simulink model "AirDirecCtrlMgr"  (R2022b)
settings: Solver=FixedStepDiscrete  StartTime=0.0  StopTime=10.0  FixedStep=0.2  RelTol=1e-3
(part 2 of 2)

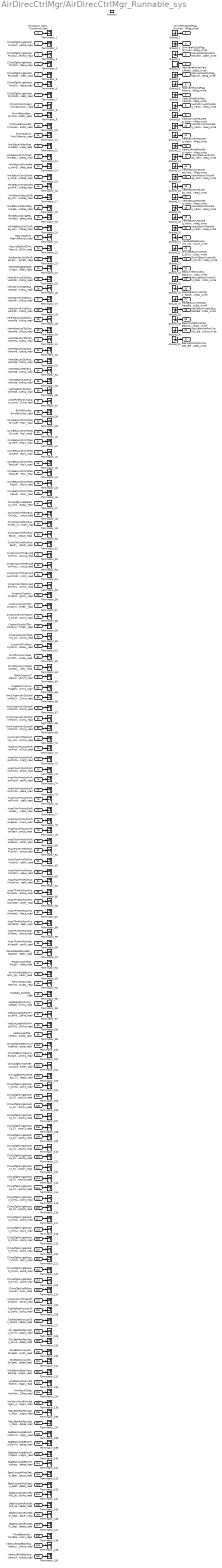
== system AirDirecCtrlMgr/AirDirecCtrlMgr_Runnable_sys ==
  ClimaActv_Ngcc_ClimaActv_read : Inport
  ClimaCfgForVgaMotorPrsntAtRowFirstLeMid_ClimaCfgForVgaMotorPrsntAtRowFirstLeMid_read : Inport  Port=2
  ClimaCfgForVgaMotorPrsntAtRowFirstRiMid_ClimaCfgForVgaMotorPrsntAtRowFirstRiMid_read : Inport  Port=3
  ClimaCfgForVgaMotorPrsntAtRowFirstSideLe_ClimaCfgForVgaMotorPrsntAtRowFirstSideLe_read : Inport  Port=4
  ClimaCfgForVgaMotorPrsntAtRowFirstSideRi_ClimaCfgForVgaMotorPrsntAtRowFirstSideRi_read : Inport  Port=5
  ClimaCfgForVgaMotorPrsntAtRowSecSideLe_ClimaCfgForVgaMotorPrsntAtRowSecSideLe_read : Inport  Port=6
  ClimaCfgForVgaMotorPrsntAtRowSecSideRi_ClimaCfgForVgaMotorPrsntAtRowSecSideRi_read : Inport  Port=7
  ClimaCtrlActvNgcc_ClimaCtrlActv_read : Inport  Port=8
  DrvrHdPosnDetn_DrvrHdPosnDetn_read : Inport  Port=9
  FrntHvacBlowerSts_FrntHvacBlowerSts_read : Inport  Port=10
  FrntVGAerror_FrntVGAerror_read : Inport  Port=11
  HmiElecAirAdpvReq_HmiElecAirAdpvReq_read : Inport  Port=12
  HmiElecAirDirCrtlReq_HmiElecAirDirCrtlReq_read : Inport  Port=13
  HmiElecAirDirModReq_HmiElecAirDirModReq_read : Inport  Port=14
  HmiElecAirDrvrSwtReq_HmiElecAirDrvrSwtReq_read : Inport  Port=15
  HmiElecAirFrntSwtReq_HmiElecAirFrntSwtReq_read : Inport  Port=16
  HmiElecAirPassSwtReq_HmiElecAirPassSwtReq_read : Inport  Port=17
  HmiElecAirReSwtReq_HmiElecAirReSwtReq_read : Inport  Port=18
  HmiElecAirSwngReq_HmiElecAirSwngReq_read : Inport  Port=19
  HmiReElecAirDirCrtlReq_HmiReElecAirDirCrtlReq_read : Inport  Port=20
  RearVGAerror_RearVGAerror_read : Inport  Port=21
  ResrvdSigForECM4_ResrvdSigForECM4_read : Inport  Port=22
  StrtRemSts1WdStrtRemSts_Ngcc_StrtRemSts1WdStrtRemSts_read : Inport  Port=23
  VehModMngtGlbSafe1_Ngcc_VehModMngtGlbSafe1_read : Inport  Port=24
  VentnDrvrLeXStsRsp_VentnDrvrLeXStsRsp_read : Inport  Port=25
  VentnDrvrLeYStsRsp_VentnDrvrLeYStsRsp_read : Inport  Port=26
  VentnDrvrRiXStsRsp_VentnDrvrRiXStsRsp_read : Inport  Port=27
  VentnDrvrRiYStsRsp_VentnDrvrRiYStsRsp_read : Inport  Port=28
  VentnPassLeXStsRsp_VentnPassLeXStsRsp_read : Inport  Port=29
  VentnPassLeYStsRsp_VentnPassLeYStsRsp_read : Inport  Port=30
  VentnPassRiXStsRsp_VentnPassRiXStsRsp_read : Inport  Port=31
  VentnPassRiYStsRsp_VentnPassRiYStsRsp_read : Inport  Port=32
  VentnSecLeXStsRsp_VentnSecLeXStsRsp_read : Inport  Port=33
  VentnSecLeYStsRsp_VentnSecLeYStsRsp_read : Inport  Port=34
  VentnSecRiXStsRsp_VentnSecRiXStsRsp_read : Inport  Port=35
  VentnSecRiYStsRsp_VentnSecRiYStsRsp_read : Inport  Port=36
  AmbTFildForClimaNgcc_AmbTFildForClima_read : Inport  Port=37
  BlwAftRunSts_BlwAftRunSts_read : Inport  Port=38
  CcmElecAirDirCrtlReqDrvrLePosX_CcmElecAirDirCrtlReqDrvrLePosX_read : Inport  Port=39
  CcmElecAirDirCrtlReqDrvrLePosY_CcmElecAirDirCrtlReqDrvrLePosY_read : Inport  Port=40
  CcmElecAirDirCrtlReqDrvrRiPosX_CcmElecAirDirCrtlReqDrvrRiPosX_read : Inport  Port=41
  CcmElecAirDirCrtlReqDrvrRiPosY_CcmElecAirDirCrtlReqDrvrRiPosY_read : Inport  Port=42
  CcmElecAirDirCrtlReqPassLePosX_CcmElecAirDirCrtlReqPassLePosX_read : Inport  Port=43
  CcmElecAirDirCrtlReqPassLePosY_CcmElecAirDirCrtlReqPassLePosY_read : Inport  Port=44
  CcmElecAirDirCrtlReqPassRiPosX_CcmElecAirDirCrtlReqPassRiPosX_read : Inport  Port=45
  CcmElecAirDirCrtlReqPassRiPosY_CcmElecAirDirCrtlReqPassRiPosY_read : Inport  Port=46
  ClimaCfgForHeadRadar_ClimaCfgForHeadRadar_read : Inport  Port=47
  ClimaTAtCmftForRowFirstLe_ClimaTAtCmftForRowFirstLe_read : Inport  Port=48
  ClimaTAtCmftForRowFirstRi_ClimaTAtCmftForRowFirstRi_read : Inport  Port=49
  ClimaTAtCmftForRowSecLe_ClimaTAtCmftForRowSecLe_read : Inport  Port=50
  ClimaTAtCmftForRowSecRi_ClimaTAtCmftForRowSecRi_read : Inport  Port=51
  CmptmtAirTCtrlErrAtRowFirstAvg_CmptmtAirTCtrlErrAtRowFirstAvg_read : Inport  Port=52
  CmptmtAirTCtrlErrAtRowFirstLe_CmptmtAirTCtrlErrAtRowFirstLe_read : Inport  Port=53
  CmptmtAirTCtrlErrAtRowFirstRi_CmptmtAirTCtrlErrAtRowFirstRi_read : Inport  Port=54
  CmptmtAirTEstimdAtRowFirst_CmptmtAirTEstimdAtRowFirst_read : Inport  Port=55
  CmptmtClngActv_CmptmtClngActv_read : Inport  Port=56
  CmptmtIntrMtrlTErr_CmptmtIntrMtrlTErr_read : Inport  Port=57
  CmptmtIntrMtrlTEstimd_CmptmtIntrMtrlTEstimd_read : Inport  Port=58
  CmptmtSunMtrlTErr_CmptmtSunMtrlTErr_read : Inport  Port=59
  CmptmtSunMtrlTEstimd_CmptmtSunMtrlTEstimd_read : Inport  Port=60
  CmptmtTFrntRaw_CmptmtTFrntRaw_read : Inport  Port=61
  DrvrPfmncAlrmReq_DrvrPfmncAlrmReq_read : Inport  Port=62
  DrvrPfmncAlrmReqFromDHU_DrvrPfmncAlrmReqFromDHU_read : Inport  Port=63
  FbForCmptmtT_FbForCmptmtT_read : Inport  Port=64
  FragStsFrmClima_FragStsFrmClima_read : Inport  Port=65
  HmiCmptmtAirDistbnFrntForClima_HmiCmptmtAirDistbnFrntForClima_read : Inport  Port=66
  HmiCmptmtAirDistbnFrntForClima_HmiCmptmtAirDistbnFrntRiForClima_read : Inport  Port=67
  HmiCmptmtAirDistbnFrntForClima_HmiCmptmtAirDistbnReForClima_read : Inport  Port=68
  HmiCmptmtAirDistbnFrntForClima_HmiCmptmtAirDistbnReRiForClima_read : Inport  Port=69
  HmiCmptmtTSpForClima_HmiCmptmtTSpForClima_read : Inport  Port=70
  HvacFlowFrntActForRowFirst_HvacFlowFrntActForRowFirst_read : Inport  Port=71
  HvacFlowFrntActForRowFirstAtVent_HvacFlowFrntActForRowFirstAtVent_read : Inport  Port=72
  HvacFlowFrntActForRowFirstAtVent_HvacFlowFrntActForRowFirstAtVentLe_read : Inport  Port=73
  HvacFlowFrntActForRowFirstAtVent_HvacFlowFrntActForRowFirstAtVentRi_read : Inport  Port=74
  HvacFlowFrntActForRowFirstAtVent_HvacFlowFrntActForRowFirstAtVentSideLe_read : Inport  Port=75
  HvacFlowFrntActForRowFirstAtVent_HvacFlowFrntActForRowFirstAtVentSideRi_read : Inport  Port=76
  HvacFlowFrntActForRowSec_HvacFlowFrntActForRowSec_read : Inport  Port=77
  HvacFlowFrntActForRowSecAtVent_HvacFlowFrntActForRowSecAtVent_read : Inport  Port=78
  HvacFlowFrntActForRowSecAtVent_HvacFlowFrntActForRowSecAtVentLe_read : Inport  Port=79
  HvacFlowFrntActForRowSecAtVent_HvacFlowFrntActForRowSecAtVentRi_read : Inport  Port=80
  HvacFlowFrntForRowFirstAtVentLe_HvacFlowFrntForRowFirstAtVentLe_read : Inport  Port=81
  HvacFlowFrntForRowFirstAtVentRi_HvacFlowFrntForRowFirstAtVentRi_read : Inport  Port=82
  HvacFlowFrntForRowFirstAtVentSideLe_HvacFlowFrntForRowFirstAtVentSideLe_read : Inport  Port=83
  HvacFlowFrntForRowFirstAtVentSideRi_HvacFlowFrntForRowFirstAtVentSideRi_read : Inport  Port=84
  HvacTFrntForRowFirstAtVentLe_HvacTFrntForRowFirstAtVentLe_read : Inport  Port=85
  HvacTFrntForRowFirstAtVentRi_HvacTFrntForRowFirstAtVentRi_read : Inport  Port=86
  HvacTFrntForRowFirstAtVentSideLe_HvacTFrntForRowFirstAtVentSideLe_read : Inport  Port=87
  HvacTFrntForRowFirstAtVentSideRi_HvacTFrntForRowFirstAtVentSideRi_read : Inport  Port=88
  HvacTFrntForRowSecAtVentLe_HvacTFrntForRowSecAtVentLe_read : Inport  Port=89
  HvacTFrntForRowSecAtVentRi_HvacTFrntForRowSecAtVentRi_read : Inport  Port=90
  PassSideHdPosnDetn_PassSideHdPosnDetn_read : Inport  Port=91
  PrkgClimaOnReq_PrkgClimaOnReq_read : Inport  Port=92
  PwrAllwdForElecAirVentn_Ngcc_PwrAllwdForElecAirVentn_read : Inport  Port=93
  RemVentActvSts_RemVentActvSts_read : Inport  Port=94
  SunData_SunData_read : Inport  Port=95
  VehSpdLgtForClima_VehSpdLgtForClima_read : Inport  Port=96
  VehSunLoadAtRowFirstLeFild_VehSunLoadAtRowFirstLeFild_read : Inport  Port=97
  VehSunLoadAtRowFirstRiFild_VehSunLoadAtRowFirstRiFild_read : Inport  Port=98
  VehSunLoadTot_VehSunLoadTot_read : Inport  Port=99
  ClimaCfgForElectricLiftingModule_ClimaCfgForElectricLiftingModule_read : Inport  Port=100
  ClimaCfgForInteriorViewCamera_ClimaCfgForInteriorViewCamera_read : Inport  Port=101
  ClimaCfgForMARKET_ClimaCfgForMARKET_read : Inport  Port=102
  ClimaCfgForPowerSeats_ClimaCfgForPowerSeats_read : Inport  Port=103
  ClimaCfgForVgaMotor1_ClimaCfgForVgaMotor1_read : Inport  Port=104
  ClimaCfgForVgaMotor10_ClimaCfgForVgaMotor10_read : Inport  Port=105
  ClimaCfgForVgaMotor11_ClimaCfgForVgaMotor11_read : Inport  Port=106
  ClimaCfgForVgaMotor12_ClimaCfgForVgaMotor12_read : Inport  Port=107
  ClimaCfgForVgaMotor13_ClimaCfgForVgaMotor13_read : Inport  Port=108
  ClimaCfgForVgaMotor14_ClimaCfgForVgaMotor14_read : Inport  Port=109
  ClimaCfgForVgaMotor15_ClimaCfgForVgaMotor15_read : Inport  Port=110
  ClimaCfgForVgaMotor16_ClimaCfgForVgaMotor16_read : Inport  Port=111
  ClimaCfgForVgaMotor17_ClimaCfgForVgaMotor17_read : Inport  Port=112
  ClimaCfgForVgaMotor18_ClimaCfgForVgaMotor18_read : Inport  Port=113
  ClimaCfgForVgaMotor19_ClimaCfgForVgaMotor19_read : Inport  Port=114
  ClimaCfgForVgaMotor2_ClimaCfgForVgaMotor2_read : Inport  Port=115
  ClimaCfgForVgaMotor20_ClimaCfgForVgaMotor20_read : Inport  Port=116
  ClimaCfgForVgaMotor3_ClimaCfgForVgaMotor3_read : Inport  Port=117
  ClimaCfgForVgaMotor4_ClimaCfgForVgaMotor4_read : Inport  Port=118
  ClimaCfgForVgaMotor5_ClimaCfgForVgaMotor5_read : Inport  Port=119
  ClimaCfgForVgaMotor6_ClimaCfgForVgaMotor6_read : Inport  Port=120
  ClimaCfgForVgaMotor7_ClimaCfgForVgaMotor7_read : Inport  Port=121
  ClimaCfgForVgaMotor8_ClimaCfgForVgaMotor8_read : Inport  Port=122
  ClimaCfgForVgaMotor9_ClimaCfgForVgaMotor9_read : Inport  Port=123
  ClimaCfgWindFollowHumanMov_ClimaCfgWindFollowHumanMov_read : Inport  Port=124
  CmptmtAirTCtrlErrQf_CmptmtAirTCtrlErrQf_read : Inport  Port=125
  CopilotSeatOccupySts_CopilotSeatOccupySts_read : Inport  Port=126
  CopilotSeatOccupySts_CopilotSeatOccupySts_IsUpdated_read : Inport  Port=127
  DrvrSeatPosPercNgcc_DrvrSeatPosPercNgcc_read : Inport  Port=128
  DrvrSeatPosPercNgcc_DrvrSeatPosPercNgcc_IsUpdated_read : Inport  Port=129
  DrvSeatOccupySts_DrvSeatOccupySts_read : Inport  Port=130
  DrvSeatOccupySts_DrvSeatOccupySts_IsUpdated_read : Inport  Port=131
  HmiElecAirBodyVentLebtnNgcc_HmiElecAirBodyVentLebtnNgcc_read : Inport  Port=132
  HmiElecAirBodyVentRibtnNgcc_HmiElecAirBodyVentRibtnNgcc_read : Inport  Port=133
  HmiMaxACReq_HmiMaxACReq_read : Inport  Port=134
  HmiSmrtWindFolwSetNgcc_HmiSmrtWindFolwSetNgcc_read : Inport  Port=135
  PassSeatPosPercNgcc_PassSeatPosPercNgcc_read : Inport  Port=136
  PassSeatPosPercNgcc_PassSeatPosPercNgcc_IsUpdated_read : Inport  Port=137
  SeatBackAngleRowFirstDrvrNgcc_SeatBackAngleRowFirstDrvrNgcc_read : Inport  Port=138
  SeatBackAngleRowFirstDrvrNgcc_SeatBackAngleRowFirstDrvrNgcc_IsUpdated_read : Inport  Port=139
  SeatBackAngleRowFirstPassNgcc_SeatBackAngleRowFirstPassNgcc_read : Inport  Port=140
  SeatBackAngleRowFirstPassNgcc_SeatBackAngleRowFirstPassNgcc_IsUpdated_read : Inport  Port=141
  SeatOccptAtRowSecLe_SeatOccptAtRowSecLe_read : Inport  Port=142
  SeatOccptAtRowSecLe_SeatOccptAtRowSecLe_IsUpdated_read : Inport  Port=143
  SeatOccptAtRowSecMid_SeatOccptAtRowSecMid_read : Inport  Port=144
  SeatOccptAtRowSecMid_SeatOccptAtRowSecMid_IsUpdated_read : Inport  Port=145
  SeatOccptAtRowSecRi_SeatOccptAtRowSecRi_read : Inport  Port=146
  SeatOccptAtRowSecRi_SeatOccptAtRowSecRi_IsUpdated_read : Inport  Port=147
  TiAndDateIndcn_TiAndDateIndcn_read : Inport  Port=148
  VentnLiftModPosnRsp_VentnLiftModPosnRsp_read : Inport  Port=149
  VentnLiftModStsRsp_VentnLiftModStsRsp_read : Inport  Port=150
  function : TriggerPort  TriggerType=function-call  VariantControl=(inherit)  SampleTimeType=periodic  SampleTime=-1
  Ground_{1..3,5..31} (30 alike) : Ground
  Ground_4 : Subsystem
  Terminator_{1..150} (150 alike) : Terminator
  DrvrVentLeClsdFlag_DrvrVentLeClsdFlag_write : Outport
      settings: EnsureOutportIsVirtual=on
  DrvrVentRiClsdFlag_DrvrVentRiClsdFlag_write : Outport  Port=2
      settings: EnsureOutportIsVirtual=on
  MemSaveFlgForCenVentLiftModErr_MemSaveFlgForCenVentLiftModErr_write : Outport  Port=3
      settings: EnsureOutportIsVirtual=on
  MemSaveForCenVentLiftModSts_MemSaveForCenVentLiftModSts_write : Outport  Port=4
      settings: EnsureOutportIsVirtual=on
  PassVentLeClsdFlag_PassVentLeClsdFlag_write : Outport  Port=5
      settings: EnsureOutportIsVirtual=on
  PassVentRiClsdFlag_PassVentRiClsdFlag_write : Outport  Port=6
      settings: EnsureOutportIsVirtual=on
  VentLiftModCaliReq_VentLiftModCaliReq_write : Outport  Port=7
      settings: EnsureOutportIsVirtual=on
  VentnDrvrLeXPsonReq_VentnDrvrLeXPsonReq_write : Outport  Port=8
      settings: EnsureOutportIsVirtual=on
  VentnDrvrLeYPsonReq_VentnDrvrLeYPsonReq_write : Outport  Port=9
      settings: EnsureOutportIsVirtual=on
  VentnDrvrRiXPsonReq_VentnDrvrRiXPsonReq_write : Outport  Port=10
      settings: EnsureOutportIsVirtual=on
  VentnDrvrRiYPsonReq_VentnDrvrRiYPsonReq_write : Outport  Port=11
      settings: EnsureOutportIsVirtual=on
  VentnLiftModPosnReq_VentnLiftModPosnReq_write : Outport  Port=12
      settings: EnsureOutportIsVirtual=on
  VentnPassLeXPsonReq_VentnPassLeXPsonReq_write : Outport  Port=13
      settings: EnsureOutportIsVirtual=on
  VentnPassLeYPsonReq_VentnPassLeYPsonReq_write : Outport  Port=14
      settings: EnsureOutportIsVirtual=on
  VentnPassRiXPsonReq_VentnPassRiXPsonReq_write : Outport  Port=15
      settings: EnsureOutportIsVirtual=on
  VentnPassRiYPsonReq_VentnPassRiYPsonReq_write : Outport  Port=16
      settings: EnsureOutportIsVirtual=on
  VentnSecLeXPosnReq_VentnSecLeXPosnReq_write : Outport  Port=17
      settings: EnsureOutportIsVirtual=on
  VentnSecLeYPosnReq_VentnSecLeYPosnReq_write : Outport  Port=18
      settings: EnsureOutportIsVirtual=on
  VentnSecRiXPosnReq_VentnSecRiXPosnReq_write : Outport  Port=19
      settings: EnsureOutportIsVirtual=on
  VentnSecRiYPosnReq_VentnSecRiYPosnReq_write : Outport  Port=20
      settings: EnsureOutportIsVirtual=on
  VGAStopDuePowerLimit_VGAStopDuePowerLimit_write : Outport  Port=21
      settings: EnsureOutportIsVirtual=on
  DrvrLeElecAirVentnSts_DrvrLeElecAirVentnSts_write : Outport  Port=22
      settings: EnsureOutportIsVirtual=on
  DrvrRiElecAirVentnSts_DrvrRiElecAirVentnSts_write : Outport  Port=23
      settings: EnsureOutportIsVirtual=on
  ElecAirVentnAvlSts_ElecAirVentnAvlSts_write : Outport  Port=24
      settings: EnsureOutportIsVirtual=on
  PassLeElecAirVentnSts_PassLeElecAirVentnSts_write : Outport  Port=25
      settings: EnsureOutportIsVirtual=on
  PassRiElecAirVentnSts_PassRiElecAirVentnSts_write : Outport  Port=26
      settings: EnsureOutportIsVirtual=on
  ReLeElecAirVentnSts_ReLeElecAirVentnSts_write : Outport  Port=27
      settings: EnsureOutportIsVirtual=on
  ReRiElecAirVentnSts_ReRiElecAirVentnSts_write : Outport  Port=28
      settings: EnsureOutportIsVirtual=on
  EleAirOutletCmpFact_EleAirOutletCmpFact_write : Outport  Port=29
      settings: EnsureOutportIsVirtual=on
  ElecVentnMotPwrCnsAct_ElecVentnMotPwrCnsAct_write : Outport  Port=30
      settings: EnsureOutportIsVirtual=on
  ElecVentnMotPwrCnsDes_ElecVentnMotPwrCnsDes_write : Outport  Port=31
      settings: EnsureOutportIsVirtual=on
  wires:
  ClimaActv_Ngcc_ClimaActv_read/1 -> Terminator_1/1
  ClimaCfgForVgaMotorPrsntAtRowFirstLeMid_ClimaCfgForVgaMotorPrsntAtRowFirstLeMid_read/1 -> Terminator_2/1
  ClimaCfgForVgaMotorPrsntAtRowFirstRiMid_ClimaCfgForVgaMotorPrsntAtRowFirstRiMid_read/1 -> Terminator_3/1
  ClimaCfgForVgaMotorPrsntAtRowFirstSideLe_ClimaCfgForVgaMotorPrsntAtRowFirstSideLe_read/1 -> Terminator_4/1
  ClimaCfgForVgaMotorPrsntAtRowFirstSideRi_ClimaCfgForVgaMotorPrsntAtRowFirstSideRi_read/1 -> Terminator_5/1
  ClimaCfgForVgaMotorPrsntAtRowSecSideLe_ClimaCfgForVgaMotorPrsntAtRowSecSideLe_read/1 -> Terminator_6/1
  ClimaCfgForVgaMotorPrsntAtRowSecSideRi_ClimaCfgForVgaMotorPrsntAtRowSecSideRi_read/1 -> Terminator_7/1
  ClimaCtrlActvNgcc_ClimaCtrlActv_read/1 -> Terminator_8/1
  DrvrHdPosnDetn_DrvrHdPosnDetn_read/1 -> Terminator_9/1
  FrntHvacBlowerSts_FrntHvacBlowerSts_read/1 -> Terminator_10/1
  FrntVGAerror_FrntVGAerror_read/1 -> Terminator_11/1
  HmiElecAirAdpvReq_HmiElecAirAdpvReq_read/1 -> Terminator_12/1
  HmiElecAirDirCrtlReq_HmiElecAirDirCrtlReq_read/1 -> Terminator_13/1
  HmiElecAirDirModReq_HmiElecAirDirModReq_read/1 -> Terminator_14/1
  HmiElecAirDrvrSwtReq_HmiElecAirDrvrSwtReq_read/1 -> Terminator_15/1
  HmiElecAirFrntSwtReq_HmiElecAirFrntSwtReq_read/1 -> Terminator_16/1
  HmiElecAirPassSwtReq_HmiElecAirPassSwtReq_read/1 -> Terminator_17/1
  HmiElecAirReSwtReq_HmiElecAirReSwtReq_read/1 -> Terminator_18/1
  HmiElecAirSwngReq_HmiElecAirSwngReq_read/1 -> Terminator_19/1
  HmiReElecAirDirCrtlReq_HmiReElecAirDirCrtlReq_read/1 -> Terminator_20/1
  RearVGAerror_RearVGAerror_read/1 -> Terminator_21/1
  ResrvdSigForECM4_ResrvdSigForECM4_read/1 -> Terminator_22/1
  StrtRemSts1WdStrtRemSts_Ngcc_StrtRemSts1WdStrtRemSts_read/1 -> Terminator_23/1
  VehModMngtGlbSafe1_Ngcc_VehModMngtGlbSafe1_read/1 -> Terminator_24/1
  VentnDrvrLeXStsRsp_VentnDrvrLeXStsRsp_read/1 -> Terminator_25/1
  VentnDrvrLeYStsRsp_VentnDrvrLeYStsRsp_read/1 -> Terminator_26/1
  VentnDrvrRiXStsRsp_VentnDrvrRiXStsRsp_read/1 -> Terminator_27/1
  VentnDrvrRiYStsRsp_VentnDrvrRiYStsRsp_read/1 -> Terminator_28/1
  VentnPassLeXStsRsp_VentnPassLeXStsRsp_read/1 -> Terminator_29/1
  VentnPassLeYStsRsp_VentnPassLeYStsRsp_read/1 -> Terminator_30/1
  VentnPassRiXStsRsp_VentnPassRiXStsRsp_read/1 -> Terminator_31/1
  VentnPassRiYStsRsp_VentnPassRiYStsRsp_read/1 -> Terminator_32/1
  VentnSecLeXStsRsp_VentnSecLeXStsRsp_read/1 -> Terminator_33/1
  VentnSecLeYStsRsp_VentnSecLeYStsRsp_read/1 -> Terminator_34/1
  VentnSecRiXStsRsp_VentnSecRiXStsRsp_read/1 -> Terminator_35/1
  VentnSecRiYStsRsp_VentnSecRiYStsRsp_read/1 -> Terminator_36/1
  AmbTFildForClimaNgcc_AmbTFildForClima_read/1 -> Terminator_37/1
  BlwAftRunSts_BlwAftRunSts_read/1 -> Terminator_38/1
  CcmElecAirDirCrtlReqDrvrLePosX_CcmElecAirDirCrtlReqDrvrLePosX_read/1 -> Terminator_39/1
  CcmElecAirDirCrtlReqDrvrLePosY_CcmElecAirDirCrtlReqDrvrLePosY_read/1 -> Terminator_40/1
  CcmElecAirDirCrtlReqDrvrRiPosX_CcmElecAirDirCrtlReqDrvrRiPosX_read/1 -> Terminator_41/1
  CcmElecAirDirCrtlReqDrvrRiPosY_CcmElecAirDirCrtlReqDrvrRiPosY_read/1 -> Terminator_42/1
  CcmElecAirDirCrtlReqPassLePosX_CcmElecAirDirCrtlReqPassLePosX_read/1 -> Terminator_43/1
  CcmElecAirDirCrtlReqPassLePosY_CcmElecAirDirCrtlReqPassLePosY_read/1 -> Terminator_44/1
  CcmElecAirDirCrtlReqPassRiPosX_CcmElecAirDirCrtlReqPassRiPosX_read/1 -> Terminator_45/1
  CcmElecAirDirCrtlReqPassRiPosY_CcmElecAirDirCrtlReqPassRiPosY_read/1 -> Terminator_46/1
  ClimaCfgForHeadRadar_ClimaCfgForHeadRadar_read/1 -> Terminator_47/1
  ClimaTAtCmftForRowFirstLe_ClimaTAtCmftForRowFirstLe_read/1 -> Terminator_48/1
  ClimaTAtCmftForRowFirstRi_ClimaTAtCmftForRowFirstRi_read/1 -> Terminator_49/1
  ClimaTAtCmftForRowSecLe_ClimaTAtCmftForRowSecLe_read/1 -> Terminator_50/1
  ClimaTAtCmftForRowSecRi_ClimaTAtCmftForRowSecRi_read/1 -> Terminator_51/1
  CmptmtAirTCtrlErrAtRowFirstAvg_CmptmtAirTCtrlErrAtRowFirstAvg_read/1 -> Terminator_52/1
  CmptmtAirTCtrlErrAtRowFirstLe_CmptmtAirTCtrlErrAtRowFirstLe_read/1 -> Terminator_53/1
  CmptmtAirTCtrlErrAtRowFirstRi_CmptmtAirTCtrlErrAtRowFirstRi_read/1 -> Terminator_54/1
  CmptmtAirTEstimdAtRowFirst_CmptmtAirTEstimdAtRowFirst_read/1 -> Terminator_55/1
  CmptmtClngActv_CmptmtClngActv_read/1 -> Terminator_56/1
  CmptmtIntrMtrlTErr_CmptmtIntrMtrlTErr_read/1 -> Terminator_57/1
  CmptmtIntrMtrlTEstimd_CmptmtIntrMtrlTEstimd_read/1 -> Terminator_58/1
  CmptmtSunMtrlTErr_CmptmtSunMtrlTErr_read/1 -> Terminator_59/1
  CmptmtSunMtrlTEstimd_CmptmtSunMtrlTEstimd_read/1 -> Terminator_60/1
  CmptmtTFrntRaw_CmptmtTFrntRaw_read/1 -> Terminator_61/1
  DrvrPfmncAlrmReq_DrvrPfmncAlrmReq_read/1 -> Terminator_62/1
  DrvrPfmncAlrmReqFromDHU_DrvrPfmncAlrmReqFromDHU_read/1 -> Terminator_63/1
  FbForCmptmtT_FbForCmptmtT_read/1 -> Terminator_64/1
  FragStsFrmClima_FragStsFrmClima_read/1 -> Terminator_65/1
  HmiCmptmtAirDistbnFrntForClima_HmiCmptmtAirDistbnFrntForClima_read/1 -> Terminator_66/1
  HmiCmptmtAirDistbnFrntForClima_HmiCmptmtAirDistbnFrntRiForClima_read/1 -> Terminator_67/1
  HmiCmptmtAirDistbnFrntForClima_HmiCmptmtAirDistbnReForClima_read/1 -> Terminator_68/1
  HmiCmptmtAirDistbnFrntForClima_HmiCmptmtAirDistbnReRiForClima_read/1 -> Terminator_69/1
  HmiCmptmtTSpForClima_HmiCmptmtTSpForClima_read/1 -> Terminator_70/1
  HvacFlowFrntActForRowFirst_HvacFlowFrntActForRowFirst_read/1 -> Terminator_71/1
  HvacFlowFrntActForRowFirstAtVent_HvacFlowFrntActForRowFirstAtVent_read/1 -> Terminator_72/1
  HvacFlowFrntActForRowFirstAtVent_HvacFlowFrntActForRowFirstAtVentLe_read/1 -> Terminator_73/1
  HvacFlowFrntActForRowFirstAtVent_HvacFlowFrntActForRowFirstAtVentRi_read/1 -> Terminator_74/1
  HvacFlowFrntActForRowFirstAtVent_HvacFlowFrntActForRowFirstAtVentSideLe_read/1 -> Terminator_75/1
  HvacFlowFrntActForRowFirstAtVent_HvacFlowFrntActForRowFirstAtVentSideRi_read/1 -> Terminator_76/1
  HvacFlowFrntActForRowSec_HvacFlowFrntActForRowSec_read/1 -> Terminator_77/1
  HvacFlowFrntActForRowSecAtVent_HvacFlowFrntActForRowSecAtVent_read/1 -> Terminator_78/1
  HvacFlowFrntActForRowSecAtVent_HvacFlowFrntActForRowSecAtVentLe_read/1 -> Terminator_79/1
  HvacFlowFrntActForRowSecAtVent_HvacFlowFrntActForRowSecAtVentRi_read/1 -> Terminator_80/1
  HvacFlowFrntForRowFirstAtVentLe_HvacFlowFrntForRowFirstAtVentLe_read/1 -> Terminator_81/1
  HvacFlowFrntForRowFirstAtVentRi_HvacFlowFrntForRowFirstAtVentRi_read/1 -> Terminator_82/1
  HvacFlowFrntForRowFirstAtVentSideLe_HvacFlowFrntForRowFirstAtVentSideLe_read/1 -> Terminator_83/1
  HvacFlowFrntForRowFirstAtVentSideRi_HvacFlowFrntForRowFirstAtVentSideRi_read/1 -> Terminator_84/1
  HvacTFrntForRowFirstAtVentLe_HvacTFrntForRowFirstAtVentLe_read/1 -> Terminator_85/1
  HvacTFrntForRowFirstAtVentRi_HvacTFrntForRowFirstAtVentRi_read/1 -> Terminator_86/1
  HvacTFrntForRowFirstAtVentSideLe_HvacTFrntForRowFirstAtVentSideLe_read/1 -> Terminator_87/1
  HvacTFrntForRowFirstAtVentSideRi_HvacTFrntForRowFirstAtVentSideRi_read/1 -> Terminator_88/1
  HvacTFrntForRowSecAtVentLe_HvacTFrntForRowSecAtVentLe_read/1 -> Terminator_89/1
  HvacTFrntForRowSecAtVentRi_HvacTFrntForRowSecAtVentRi_read/1 -> Terminator_90/1
  PassSideHdPosnDetn_PassSideHdPosnDetn_read/1 -> Terminator_91/1
  PrkgClimaOnReq_PrkgClimaOnReq_read/1 -> Terminator_92/1
  PwrAllwdForElecAirVentn_Ngcc_PwrAllwdForElecAirVentn_read/1 -> Terminator_93/1
  RemVentActvSts_RemVentActvSts_read/1 -> Terminator_94/1
  SunData_SunData_read/1 -> Terminator_95/1
  VehSpdLgtForClima_VehSpdLgtForClima_read/1 -> Terminator_96/1
  VehSunLoadAtRowFirstLeFild_VehSunLoadAtRowFirstLeFild_read/1 -> Terminator_97/1
  VehSunLoadAtRowFirstRiFild_VehSunLoadAtRowFirstRiFild_read/1 -> Terminator_98/1
  VehSunLoadTot_VehSunLoadTot_read/1 -> Terminator_99/1
  ClimaCfgForElectricLiftingModule_ClimaCfgForElectricLiftingModule_read/1 -> Terminator_100/1
  ClimaCfgForInteriorViewCamera_ClimaCfgForInteriorViewCamera_read/1 -> Terminator_101/1
  ClimaCfgForMARKET_ClimaCfgForMARKET_read/1 -> Terminator_102/1
  ClimaCfgForPowerSeats_ClimaCfgForPowerSeats_read/1 -> Terminator_103/1
  ClimaCfgForVgaMotor1_ClimaCfgForVgaMotor1_read/1 -> Terminator_104/1
  ClimaCfgForVgaMotor10_ClimaCfgForVgaMotor10_read/1 -> Terminator_105/1
  ClimaCfgForVgaMotor11_ClimaCfgForVgaMotor11_read/1 -> Terminator_106/1
  ClimaCfgForVgaMotor12_ClimaCfgForVgaMotor12_read/1 -> Terminator_107/1
  ClimaCfgForVgaMotor13_ClimaCfgForVgaMotor13_read/1 -> Terminator_108/1
  ClimaCfgForVgaMotor14_ClimaCfgForVgaMotor14_read/1 -> Terminator_109/1
  ClimaCfgForVgaMotor15_ClimaCfgForVgaMotor15_read/1 -> Terminator_110/1
  ClimaCfgForVgaMotor16_ClimaCfgForVgaMotor16_read/1 -> Terminator_111/1
  ClimaCfgForVgaMotor17_ClimaCfgForVgaMotor17_read/1 -> Terminator_112/1
  ClimaCfgForVgaMotor18_ClimaCfgForVgaMotor18_read/1 -> Terminator_113/1
  ClimaCfgForVgaMotor19_ClimaCfgForVgaMotor19_read/1 -> Terminator_114/1
  ClimaCfgForVgaMotor2_ClimaCfgForVgaMotor2_read/1 -> Terminator_115/1
  ClimaCfgForVgaMotor20_ClimaCfgForVgaMotor20_read/1 -> Terminator_116/1
  ClimaCfgForVgaMotor3_ClimaCfgForVgaMotor3_read/1 -> Terminator_117/1
  ClimaCfgForVgaMotor4_ClimaCfgForVgaMotor4_read/1 -> Terminator_118/1
  ClimaCfgForVgaMotor5_ClimaCfgForVgaMotor5_read/1 -> Terminator_119/1
  ClimaCfgForVgaMotor6_ClimaCfgForVgaMotor6_read/1 -> Terminator_120/1
  ClimaCfgForVgaMotor7_ClimaCfgForVgaMotor7_read/1 -> Terminator_121/1
  ClimaCfgForVgaMotor8_ClimaCfgForVgaMotor8_read/1 -> Terminator_122/1
  ClimaCfgForVgaMotor9_ClimaCfgForVgaMotor9_read/1 -> Terminator_123/1
  ClimaCfgWindFollowHumanMov_ClimaCfgWindFollowHumanMov_read/1 -> Terminator_124/1
  CmptmtAirTCtrlErrQf_CmptmtAirTCtrlErrQf_read/1 -> Terminator_125/1
  CopilotSeatOccupySts_CopilotSeatOccupySts_read/1 -> Terminator_126/1
  CopilotSeatOccupySts_CopilotSeatOccupySts_IsUpdated_read/1 -> Terminator_127/1
  DrvrSeatPosPercNgcc_DrvrSeatPosPercNgcc_read/1 -> Terminator_128/1
  DrvrSeatPosPercNgcc_DrvrSeatPosPercNgcc_IsUpdated_read/1 -> Terminator_129/1
  DrvSeatOccupySts_DrvSeatOccupySts_read/1 -> Terminator_130/1
  DrvSeatOccupySts_DrvSeatOccupySts_IsUpdated_read/1 -> Terminator_131/1
  HmiElecAirBodyVentLebtnNgcc_HmiElecAirBodyVentLebtnNgcc_read/1 -> Terminator_132/1
  HmiElecAirBodyVentRibtnNgcc_HmiElecAirBodyVentRibtnNgcc_read/1 -> Terminator_133/1
  HmiMaxACReq_HmiMaxACReq_read/1 -> Terminator_134/1
  HmiSmrtWindFolwSetNgcc_HmiSmrtWindFolwSetNgcc_read/1 -> Terminator_135/1
  PassSeatPosPercNgcc_PassSeatPosPercNgcc_read/1 -> Terminator_136/1
  PassSeatPosPercNgcc_PassSeatPosPercNgcc_IsUpdated_read/1 -> Terminator_137/1
  SeatBackAngleRowFirstDrvrNgcc_SeatBackAngleRowFirstDrvrNgcc_read/1 -> Terminator_138/1
  SeatBackAngleRowFirstDrvrNgcc_SeatBackAngleRowFirstDrvrNgcc_IsUpdated_read/1 -> Terminator_139/1
  SeatBackAngleRowFirstPassNgcc_SeatBackAngleRowFirstPassNgcc_read/1 -> Terminator_140/1
  SeatBackAngleRowFirstPassNgcc_SeatBackAngleRowFirstPassNgcc_IsUpdated_read/1 -> Terminator_141/1
  SeatOccptAtRowSecLe_SeatOccptAtRowSecLe_read/1 -> Terminator_142/1
  SeatOccptAtRowSecLe_SeatOccptAtRowSecLe_IsUpdated_read/1 -> Terminator_143/1
  SeatOccptAtRowSecMid_SeatOccptAtRowSecMid_read/1 -> Terminator_144/1
  SeatOccptAtRowSecMid_SeatOccptAtRowSecMid_IsUpdated_read/1 -> Terminator_145/1
  SeatOccptAtRowSecRi_SeatOccptAtRowSecRi_read/1 -> Terminator_146/1
  SeatOccptAtRowSecRi_SeatOccptAtRowSecRi_IsUpdated_read/1 -> Terminator_147/1
  TiAndDateIndcn_TiAndDateIndcn_read/1 -> Terminator_148/1
  VentnLiftModPosnRsp_VentnLiftModPosnRsp_read/1 -> Terminator_149/1
  VentnLiftModStsRsp_VentnLiftModStsRsp_read/1 -> Terminator_150/1
  Ground_1/1 -> DrvrVentLeClsdFlag_DrvrVentLeClsdFlag_write/1
  Ground_2/1 -> DrvrVentRiClsdFlag_DrvrVentRiClsdFlag_write/1
  Ground_3/1 -> MemSaveFlgForCenVentLiftModErr_MemSaveFlgForCenVentLiftModErr_write/1
  Ground_4/1(Out1) -> MemSaveForCenVentLiftModSts_MemSaveForCenVentLiftModSts_write/1
  Ground_5/1 -> PassVentLeClsdFlag_PassVentLeClsdFlag_write/1
  Ground_6/1 -> PassVentRiClsdFlag_PassVentRiClsdFlag_write/1
  Ground_7/1 -> VentLiftModCaliReq_VentLiftModCaliReq_write/1
  Ground_8/1 -> VentnDrvrLeXPsonReq_VentnDrvrLeXPsonReq_write/1
  Ground_9/1 -> VentnDrvrLeYPsonReq_VentnDrvrLeYPsonReq_write/1
  Ground_10/1 -> VentnDrvrRiXPsonReq_VentnDrvrRiXPsonReq_write/1
  Ground_11/1 -> VentnDrvrRiYPsonReq_VentnDrvrRiYPsonReq_write/1
  Ground_12/1 -> VentnLiftModPosnReq_VentnLiftModPosnReq_write/1
  Ground_13/1 -> VentnPassLeXPsonReq_VentnPassLeXPsonReq_write/1
  Ground_14/1 -> VentnPassLeYPsonReq_VentnPassLeYPsonReq_write/1
  Ground_15/1 -> VentnPassRiXPsonReq_VentnPassRiXPsonReq_write/1
  Ground_16/1 -> VentnPassRiYPsonReq_VentnPassRiYPsonReq_write/1
  Ground_17/1 -> VentnSecLeXPosnReq_VentnSecLeXPosnReq_write/1
  Ground_18/1 -> VentnSecLeYPosnReq_VentnSecLeYPosnReq_write/1
  Ground_19/1 -> VentnSecRiXPosnReq_VentnSecRiXPosnReq_write/1
  Ground_20/1 -> VentnSecRiYPosnReq_VentnSecRiYPosnReq_write/1
  Ground_21/1 -> VGAStopDuePowerLimit_VGAStopDuePowerLimit_write/1
  Ground_22/1 -> DrvrLeElecAirVentnSts_DrvrLeElecAirVentnSts_write/1
  Ground_23/1 -> DrvrRiElecAirVentnSts_DrvrRiElecAirVentnSts_write/1
  Ground_24/1 -> ElecAirVentnAvlSts_ElecAirVentnAvlSts_write/1
  Ground_25/1 -> PassLeElecAirVentnSts_PassLeElecAirVentnSts_write/1
  Ground_26/1 -> PassRiElecAirVentnSts_PassRiElecAirVentnSts_write/1
  Ground_27/1 -> ReLeElecAirVentnSts_ReLeElecAirVentnSts_write/1
  Ground_28/1 -> ReRiElecAirVentnSts_ReRiElecAirVentnSts_write/1
  Ground_29/1 -> EleAirOutletCmpFact_EleAirOutletCmpFact_write/1
  Ground_30/1 -> ElecVentnMotPwrCnsAct_ElecVentnMotPwrCnsAct_write/1
  Ground_31/1 -> ElecVentnMotPwrCnsDes_ElecVentnMotPwrCnsDes_write/1

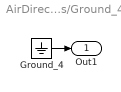
== system AirDirecCtrlMgr/AirDirecCtrlMgr_Runnable_sys/Ground_4 ==
  Ground_4 : Ground
  Out1 : Outport  OutDataTypeStr=Bus: MemSaveLiftMod  PortDimensions=1
  wires:
  Ground_4/1 -> Out1/1
